simulink model "TosAeb"  (R2021a)
settings: Solver=FixedStepDiscrete  StartTime=0.0  StopTime=inf  FixedStep=0.01  RelTol=1e-3
undefined symbols (not defined in the file or in its folder): ACCModSts, AccSts1, AEB_ObjectMovingOrientation, AEB_ObjectMovingState, CN_BTCActive, CN_CurrentGearR, CN_CurrentGearR_DHT, CN_PTCActive, CN_VDCActive, CN_VSC_EspOff, CN_VSC_FCW_AEB_Error, CN_VSC_FCW_AEB_NoDispay, CN_VSC_FCW_AEB_PerfmDgdtn, CN_VSC_FCW_AEB_TempUnAvlbl, CN_VSC_VehStandstill, CNaccelAvoidMax, CNaccelAvoidMin, CNbrakePedalPress, CNcomfortStrLeftAccelMax, CNcomfortStrLeftAccelMin, CNcomfortStrRightAccelMax, CNcomfortStrRightAccelMin, CNDrvDoorOpen, CNDrvModeEco, CNDrvModeNorm, CNDrvModeOther, CNDrvModeSport, CNDrvSBRreminder, CNNull, CNobjInvalidID, CNPassDoorOpen, CNPassSBRreminder, CNPEBSAccelNegInf, CNPEBSAccelNull, CNPEBSTimeInf, CNPEBSTimeNull, CNSTOPMAX, CNSTOPNULL, CNsubjWidth, CNSwtOFF, CNSwtON, CNTTCMAX, CNTTCNULL, CNVehConfigAEBCycNone, CNVehConfigAEBNone, CNVehConfigAEBPedNone, CNVehConfigFCWNone, CNVehConfigJANone, CollisionSeverity, FALSE, FCWSnvty, JACycObjCalcInfoDefaultParams, JAPedObjCalcInfoDefaultParams, JAVehObjCalcInfoDefaultParams, latCycObjCalcInfoDefaultParams, latPedObjCalcInfoDefaultParams, longObjCalcInfoDefaultParams, ModSts, movingStatus, ObjectType ... (+1112)
libraries: simulink x3031, AEBSFunctionLib x50, Ta_Library x33, AEBSStateMachineLib x23, Mylibrary x12, simulink_extras x7, ObjSelect_lib x3, dspstat3 x2
(part 3 of 102)

== system TosAeb/FcwAebJa_Step_sys/ALG_AEBSystem/ALG_AEBOutput ==
  DiaManageInfo : Inport  OutDataTypeStr=Bus: BusDiagManageInfo
  VehCanInfo : Inport  Port=2
  vehConfigInfoOut : Inport  Port=3
  latPedCalcInfo : Inport  Port=4  OutDataTypeStr=Bus: BusLatPedObjCalcInfo
  latCycCalcInfo : Inport  Port=5  OutDataTypeStr=Bus: BusLatCycObjCalcInfo
  AEBSFuncOut : Inport  Port=6
  vehStateCalcInfo : Inport  Port=7
  longObjCalcInfo : Inport  Port=8
  AEBModStsFuncImpArb : Inport  Port=9
  AEBModRawSts : Inport  Port=10  OutDataTypeStr=Bus: BusAEBModRawSts
  latPedTgtCalcInfo : Inport  Port=11
  latCycTgtCalcInfo : Inport  Port=12
  AEBJAPedTgtCalcInfo : Inport  Port=13
  JAVehTgtCalcInfo : Inport  Port=14
  FCWSnvtyALGInfo : Inport  Port=15
  AEBS_BrkInfoCalc : Subsystem  TreatAsAtomicUnit=on
  AEBS_DVRInfoCalc : Subsystem  TreatAsAtomicUnit=on
  AEBS_IPHUTInfoCalc : Subsystem  TreatAsAtomicUnit=on
  AEB_EDR_JAPED_INFOMap : Subsystem  TreatAsAtomicUnit=on
  AEB_EDR_JAVEH_INFOMap : Subsystem  TreatAsAtomicUnit=on
  AEB_EDR_LATCYC_INFOMap : Subsystem  TreatAsAtomicUnit=on
  AEB_EDR_LATPED_INFOMap : Subsystem  TreatAsAtomicUnit=on
  AEB_EDR_LONOBJ_INFOMap : Subsystem  TreatAsAtomicUnit=on
  AEB_FUNC_STS_INFOMap : Subsystem  TreatAsAtomicUnit=on
  Bus Creator : BusCreator  Inputs=12
  Bus Selector : BusSelector  OutputSignals=AEBSFuncOut.ABALvl,AEBSFuncOut.ABATrigger,AEBModStsFuncImpArb.brkFuncImpArb.EBAFuncImpArb,AEBModRawSts.vehRawModSts.vehAEBpartRawModSts,AEBModRawSts.vehRawModSts.vehAEBfullRawModSts,AEBModRawSts.vehRawModSts.vehEBARawModSts,AEBModRawSts.latAEBRawModSts.pedAEBfullRawModSts,AEBModRawSts.latAEBRawModSts.cycAEBpartRawModSts,AEBModRawSts.latAEBRawModSts.cycAEBfullRawModSts,AEBModRawSts.JA_AEBRawModSts.JA_vehAEBRawModSts,AEBModRawSts.JA_AEBRawModSts.JA_pedAEBRawModSts,AEBSFuncOut.AEBTrigger,AEBSFuncOut.axTgtReqValue,AEBSFuncOut.acuteWarningTrigger,AEBSFuncOut.acuteWarningLvl,AEBSFuncOut.ABPTrigger,AEBModStsFuncImpArb.brkFuncImpArb.AEBpartFuncImpArb,AEBModStsFuncImpArb.brkFuncImpArb.AEBfullFuncImpArb,AEBModStsFuncImpArb.brkFuncImpArb.cycAEBpartFuncImpArb,AEBModStsFuncImpArb.brkFuncImpArb.cycAEBfullFuncImpArb,AEBModStsFuncImpArb.brkFuncImpArb.pedAEBfullFuncImpArb,AEBModStsFuncImpArb.brkFuncImpArb.JA_AEBfullFuncImpArb,AEBModStsFuncImpArb.brkFuncImpArb.FCTBFuncImpArb,AEBModRawSts.JA_AEBRawModSts.JA_cycAEBRawModSts  OutputAsBus=on
  Bus Selector1 : BusSelector  OutputSignals=vehConfigInfo.VehicleConfigFCW,vehConfigInfo.VehicleConfigAEB,longObjCalcInfo,vehConfigInfo.VehicleConfigAEBPed,latPedTgtCalcInfo,vehConfigInfo.VehicleConfigAEBCyc,latCycTgtCalcInfo,JAVehTgtCalcInfo,vehConfigInfo.VehConfigAEBJA,AEBJAPedTgtCalcInfo
  Bus Selector2 : BusSelector  OutputSignals=vehConfigInfo.VehicleConfigFCW,vehConfigInfo.VehicleConfigAEB,longObjCalcInfo
  Bus Selector3 : BusSelector  OutputSignals=vehConfigInfo.VehicleConfigAEBPed,latPedTgtCalcInfo
  Bus Selector4 : BusSelector  OutputSignals=vehConfigInfo.VehicleConfigAEBCyc,latCycTgtCalcInfo
  Bus Selector5 : BusSelector  OutputSignals=vehConfigInfo.VehConfigAEBJA,AEBJAPedTgtCalcInfo
  Bus Selector6 : BusSelector  OutputSignals=vehConfigInfo.VehConfigAEBJA,JAVehTgtCalcInfo
  Bus Selector7 : BusSelector  OutputSignals=AEBModStsFuncImpArb
  AEB_BRK_INFO : Outport  OutDataTypeStr=Bus: AEB_BRK_INFO_BUS
  AEB_DVR_INFO : Outport  Port=2  OutDataTypeStr=Bus: AEB_DVR_INFO_BUS
  AEB_IPHUT_INFO : Outport  Port=3  OutDataTypeStr=Bus: AEB_IPHUT_INFO_BUS
  AEB_EDR_LONOBJ_INFO : Outport  Port=4  OutDataTypeStr=Bus: AEB_EDR_LONOBJ_INFO_BUS
  AEB_EDR_LATPED_INFO : Outport  Port=5  OutDataTypeStr=Bus: AEB_EDR_LATPED_INFO_BUS
  AEB_EDR_LATCYC_INFO : Outport  Port=6  OutDataTypeStr=Bus: AEB_EDR_LATCYC_INFO_BUS
  AEB_EDR_JAPED_INFO : Outport  Port=7  OutDataTypeStr=Bus: AEB_EDR_JAPED_INFO_BUS
  AEB_EDR_JAVEH_INFO : Outport  Port=8  OutDataTypeStr=Bus: AEB_EDR_JAVEH_INFO_BUS
  AEB_FUNC_STS_INFO : Outport  Port=9  OutDataTypeStr=Bus: AEB_FUNC_STS_INFO_BUS
  wires:
  Bus Selector4/2 -> AEB_EDR_LATCYC_INFOMap/2(latCycTgtCalcInfo)  "<latCycTgtCalcInfo>"
  AEBSFuncOut/1 -> Bus Creator/4  "AEBSFuncOut"
  AEBJAPedTgtCalcInfo/1 -> Bus Creator/11  "AEBJAPedTgtCalcInfo"
  AEBModStsFuncImpArb/1 -> Bus Creator/8  "AEBModStsFuncImpArb"
  VehCanInfo/1 -> Bus Creator/2  "VehCanInfo"
  AEB_EDR_LATCYC_INFOMap/1(AEB_EDR_LATCYC_INFO) -> AEB_EDR_LATCYC_INFO/1
  JAVehTgtCalcInfo/1 -> Bus Creator/12  "JAVehTgtCalcInfo"
  latCycTgtCalcInfo/1 -> Bus Creator/10  "latCycTgtCalcInfo"
  Bus Selector1/1 -> AEBS_DVRInfoCalc/1(VehicleConfigFCW)  "<VehicleConfigFCW>"
  Bus Selector/1 -> AEBS_BrkInfoCalc/1(brkInfoInputSignals)
  Bus Selector1/8 -> AEBS_DVRInfoCalc/8(JAVehTgtCalcInfo)  "<JAVehTgtCalcInfo>"
  Bus Selector2/1 -> AEB_EDR_LONOBJ_INFOMap/1(VehicleConfigFCW)  "<VehicleConfigFCW>"
  AEB_FUNC_STS_INFOMap/1(AEB_FUNC_STS_INFO) -> AEB_FUNC_STS_INFO/1
  Bus Selector1/3 -> AEBS_DVRInfoCalc/3(longObjCalcInfo)  "<longObjCalcInfo>"
  latPedCalcInfo/1 -> AEBS_IPHUTInfoCalc/2(latPedCalcInfo)  "latPedCalcInfo"
  Bus Selector1/9 -> AEBS_DVRInfoCalc/9(VehConfigAEBJA)  "<VehConfigAEBJA>"
  Bus Selector7/1 -> AEB_FUNC_STS_INFOMap/1(AEBModStsFuncImpArb)  "<AEBModStsFuncImpArb>"
  vehStateCalcInfo/1 -> Bus Creator/5  "vehStateCalcInfo"
  Bus Creator/1 -> Bus Selector/1, Bus Selector1/1, Bus Selector2/1, Bus Selector3/1, Bus Selector4/1, Bus Selector5/1, Bus Selector6/1, Bus Selector7/1, AEBS_IPHUTInfoCalc/1(inputSignals)
  latPedTgtCalcInfo/1 -> Bus Creator/9  "latPedTgtCalcInfo"
  Bus Selector1/10 -> AEBS_DVRInfoCalc/10(AEBJAPedTgtCalcInfo)  "<AEBJAPedTgtCalcInfo>"
  Bus Selector2/2 -> AEB_EDR_LONOBJ_INFOMap/2(VehicleConfigAEB)  "<VehicleConfigAEB>"
  vehConfigInfoOut/1 -> Bus Creator/3  "vehConfigInfo"
  latCycCalcInfo/1 -> AEBS_IPHUTInfoCalc/3(latCycCalcInfo)  "latCycCalcInfo"
  AEBModRawSts/1 -> Bus Creator/7  "AEBModRawSts"
  longObjCalcInfo/1 -> Bus Creator/6  "longObjCalcInfo"
  AEBS_BrkInfoCalc/1(AEB_BRK_INFO) -> AEB_BRK_INFO/1
  AEB_EDR_LATPED_INFOMap/1(AEB_EDR_LATPED_INFO) -> AEB_EDR_LATPED_INFO/1
  Bus Selector5/2 -> AEB_EDR_JAPED_INFOMap/2(JaPedTgtCalcInfo)  "<AEBJAPedTgtCalcInfo>"
  AEB_EDR_LONOBJ_INFOMap/1(AEB_EDR_LONOBJ_INFO) -> AEB_EDR_LONOBJ_INFO/1
  AEB_EDR_JAVEH_INFOMap/1(AEB_EDR_JAVEH_INFO) -> AEB_EDR_JAVEH_INFO/1
  Bus Selector1/4 -> AEBS_DVRInfoCalc/4(VehicleConfigAEBPed)  "<VehicleConfigAEBPed>"
  Bus Selector1/5 -> AEBS_DVRInfoCalc/5(latPedTgtCalcInfo)  "<latPedTgtCalcInfo>"
  Bus Selector1/7 -> AEBS_DVRInfoCalc/7(latCycTgtCalcInfo)  "<latCycTgtCalcInfo>"
  Bus Selector2/3 -> AEB_EDR_LONOBJ_INFOMap/3(longObjCalcInfo)  "<longObjCalcInfo>"
  AEBS_IPHUTInfoCalc/1(AEB_IPHUT_INFO) -> AEB_IPHUT_INFO/1
  Bus Selector6/1 -> AEB_EDR_JAVEH_INFOMap/1(VehConfigAEBJA)  "<VehConfigAEBJA>"
  DiaManageInfo/1 -> Bus Creator/1  "DiaManageInfo"
  Bus Selector5/1 -> AEB_EDR_JAPED_INFOMap/1(VehConfigAEBJA)  "<VehConfigAEBJA>"
  Bus Selector4/1 -> AEB_EDR_LATCYC_INFOMap/1(VehicleConfigAEBCyc)  "<VehicleConfigAEBCyc>"
  Bus Selector3/2 -> AEB_EDR_LATPED_INFOMap/2(latPedTgtCalcInfo)  "<latPedTgtCalcInfo>"
  Bus Selector6/2 -> AEB_EDR_JAVEH_INFOMap/2(JaVehTgtCalcInfo)  "<JAVehTgtCalcInfo>"
  AEBS_DVRInfoCalc/1(AEB_DVR_INFO) -> AEB_DVR_INFO/1
  Bus Selector3/1 -> AEB_EDR_LATPED_INFOMap/1(VehicleConfigAEBPed)  "<VehicleConfigAEBPed>"
  AEB_EDR_JAPED_INFOMap/1(AEB_EDR_JAPED_INFO) -> AEB_EDR_JAPED_INFO/1
  Bus Selector1/6 -> AEBS_DVRInfoCalc/6(VehicleConfigAEBCyc)  "<VehicleConfigAEBCyc>"
  Bus Selector1/2 -> AEBS_DVRInfoCalc/2(VehicleConfigAEB)  "<VehicleConfigAEB>"
  FCWSnvtyALGInfo/1 -> AEBS_IPHUTInfoCalc/4(FCWSnvtyALGInfo)  "JAVehTgtCalcInfo"

== system TosAeb/AEB_Objsel/TA_InfoRebuild/TAObjInfoCalc/For Each Subsystem ==
  IDForEach : Inport  Partition=on  PartitionDimension=1  PartitionWidth=1  PartitionOffset=0
  Cam_laneLeftPortion : Inport  Port=2
  Cam_laneAssociateConf : Inport  Port=3
  Cam_laneRightPortion : Inport  Port=4
  Cam_laneLeftConf : Inport  Port=5
  Cam_laneRightConf : Inport  Port=6
  Cam_objIntention : Inport  Port=7
  Cam_objIntentionProb : Inport  Port=8
  Cam_laneAssign : Inport  Port=9
  Cam_ObsPosX_s : Inport  Port=10
  Cam_ObsPosY_s : Inport  Port=11
  Cam_ObsAccY_s : Inport  Port=12
  Cam_ObsAccX_s : Inport  Port=13
  Cam_ObsVelY_s : Inport  Port=14
  Cam_ObsVelX_s : Inport  Port=15
  Cam_ObsID_u8 : Inport  Port=16
  Cam_ObsValid_u8 : Inport  Port=17
  Road_Info : Inport  Port=18
  For Each : ForEach  DisableCoverage=on  ShowIterationIndex=on
  ForEachInput : Subsystem
  Info_rebuild : Subsystem
  Terminator : Terminator
  vxRel : Outport  ConcatenationDimension=1
  vyRel : Outport  Port=2  ConcatenationDimension=1
  vxRelStd : Outport  Port=3  ConcatenationDimension=1
  vyRelStd : Outport  Port=4  ConcatenationDimension=1
  axRel : Outport  Port=5  ConcatenationDimension=1
  ayRel : Outport  Port=6  ConcatenationDimension=1
  axRelStd : Outport  Port=7  ConcatenationDimension=1
  ayRelStd : Outport  Port=8  ConcatenationDimension=1
  azimuthAngle : Outport  Port=9  ConcatenationDimension=1
  azimuthAngleStd : Outport  Port=10  ConcatenationDimension=1
  objLatDist2LaneCenter : Outport  Port=11  ConcatenationDimension=1
  objLatDist2EgoCenter : Outport  Port=12  ConcatenationDimension=1
  objPosition : Outport  Port=13  ConcatenationDimension=1
  wires:
  Cam_objIntentionProb/1 -> ForEachInput/8(Cam_objIntentionProb)
  Cam_laneRightConf/1 -> ForEachInput/6(Cam_laneRightConf)
  IDForEach/1 -> Terminator/1
  Info_rebuild/12(objLatDist2EgoCenter) -> objLatDist2EgoCenter/1
  Info_rebuild/10(azimuthAngleStd) -> azimuthAngleStd/1
  For Each/1 -> ForEachInput/1(Index)
  Cam_laneLeftPortion/1 -> ForEachInput/2(Cam_laneLeftPortion)
  Cam_laneAssociateConf/1 -> ForEachInput/3(Cam_laneAssociateConf)
  Cam_laneAssign/1 -> ForEachInput/9(Cam_laneAssign)
  Cam_objIntention/1 -> ForEachInput/7(Cam_objIntention)
  Cam_laneRightPortion/1 -> ForEachInput/4(Cam_laneRightPortion)
  Cam_laneLeftConf/1 -> ForEachInput/5(Cam_laneLeftConf)
  Cam_ObsPosX_s/1 -> ForEachInput/10(Cam_ObsPosX_s)
  Cam_ObsPosY_s/1 -> ForEachInput/11(Cam_ObsPosY_s)
  Cam_ObsAccY_s/1 -> ForEachInput/12(Cam_ObsAccY_s)
  Cam_ObsAccX_s/1 -> ForEachInput/13(Cam_ObsAccX_s)
  Cam_ObsVelY_s/1 -> ForEachInput/14(Cam_ObsVelY_s)
  Cam_ObsVelX_s/1 -> ForEachInput/15(Cam_ObsVelX_s)
  Info_rebuild/11(objLatDist2LaneCenter) -> objLatDist2LaneCenter/1
  ForEachInput/1(Obj_Infomation) -> Info_rebuild/1(Obj_Infomation)
  Road_Info/1 -> Info_rebuild/2(Road_Info)
  Info_rebuild/1(vxRel) -> vxRel/1
  Info_rebuild/4(vyRelStd) -> vyRelStd/1
  Info_rebuild/2(vyRel) -> vyRel/1
  Info_rebuild/3(vxRelStd) -> vxRelStd/1
  Info_rebuild/7(axRelStd) -> axRelStd/1
  Info_rebuild/5(axRel) -> axRel/1
  Info_rebuild/6(ayRel) -> ayRel/1
  Info_rebuild/8(ayRelStd) -> ayRelStd/1
  Info_rebuild/9(azimuthAngle) -> azimuthAngle/1
  Info_rebuild/13(objPosition) -> objPosition/1
  Cam_ObsValid_u8/1 -> ForEachInput/17(Cam_ObsValid_u8)
  Cam_ObsID_u8/1 -> ForEachInput/16(Cam_ObsID_u8)

== system TosAeb/AEB_Objsel/TA_InfoRebuild/TAObjInfoCalc/Signal_Rebuild ==
  vxRel : Inport
  vyRel : Inport  Port=2
  vxRelStd : Inport  Port=3
  vyRelStd : Inport  Port=4
  axRel : Inport  Port=5
  ayRel : Inport  Port=6
  axRelStd : Inport  Port=7
  ayRelStd : Inport  Port=8
  azimuthAngle : Inport  Port=9
  azimuthAngleStd : Inport  Port=10
  objLatDist2LaneCenter : Inport  Port=11
  objLatDist2EgoCenter : Inport  Port=12
  objPosition : Inport  Port=13
  Bus Creator : BusCreator  Inputs=13  OutDataTypeStr=Bus: Objrebuild_Bus  NonVirtualBus=on
  Objrebuild_Struct : Outport
  wires:
  vxRel/1 -> Bus Creator/1  "vxRel"
  vxRelStd/1 -> Bus Creator/3  "vxRelStd"
  vyRel/1 -> Bus Creator/2  "vyRel"
  axRel/1 -> Bus Creator/5  "axRel"
  ayRel/1 -> Bus Creator/6  "ayRel"
  vyRelStd/1 -> Bus Creator/4  "vyRelStd"
  azimuthAngleStd/1 -> Bus Creator/10  "azimuthAngleStd"
  ayRelStd/1 -> Bus Creator/8  "ayRelStd"
  azimuthAngle/1 -> Bus Creator/9  "azimuthAngle"
  axRelStd/1 -> Bus Creator/7  "axRelStd"
  objLatDist2LaneCenter/1 -> Bus Creator/11  "objLatDist2LaneCenter"
  objLatDist2EgoCenter/1 -> Bus Creator/12  "objLatDist2EgoCenter"
  objPosition/1 -> Bus Creator/13  "objPosition"
  Bus Creator/1 -> Objrebuild_Struct/1

== system TosAeb/AEB_Objsel/TA_Processor/AEB_ObjSelect/TargetInfoCheck ==
  TA_ObjSumInfo : Inport
  VehSelfData : Inport  Port=2
  PathEstimn : Inport  Port=3
  AccTgtIdn : Inport  Port=4
  Data_precess : Subsystem  TreatAsAtomicUnit=on
  Each_Set : Subsystem
  Signal Conversion : SignalConversion  OverrideOpt=off
  Signal Conversion1 : SignalConversion  OverrideOpt=off
  Signal Conversion2 : SignalConversion  OverrideOpt=off
  Terminator : Terminator
  Terminator1 : Terminator
  Terminator2 : Terminator
  TA_ObjSumInfo_P : Outport
  VehSelfData_P : Outport  Port=2
  AccTgtIdn_P : Outport  Port=3
  Boudingbox_Calculate : Outport  Port=4
  Obj_Coef : Outport  Port=5
  TTI : Outport  Port=6
  LatObj_Valid : Outport  Port=7
  Lat_ObjIntersect : Outport  Port=8
  Lat_TTR : Outport  Port=9
  Overlap_Flg : Outport  Port=10
  JA_TTRAssign : Outport  Port=11
  wires:
  Each_Set/1(TA_ObjID) -> Data_precess/1(TA_ObjID)  "Meas_ObjID"
  TA_ObjSumInfo/1 -> Each_Set/1(TA_ObjSumInfo), [TA_ObjSumInfo]
  Each_Set/15(TA_ObjVxAbs) -> Data_precess/15(TA_ObjVxAbs)  "Meas_ObjVxabs"
  VehSelfData/1 -> [VehSelfData], Data_precess/29(VehSelfData)
  Each_Set/10(TA_ObjDyStd) -> Data_precess/10(TA_ObjDyStd)  "Meas_ObjDyStd"
  Each_Set/24(TA_ObjConfidence) -> Data_precess/24(TA_ObjConfidence)  "Meas_ObjConfidence"
  Each_Set/13(TA_ObjVxStd) -> Data_precess/13(TA_ObjVxStd)  "Meas_ObjVxStd"
  Each_Set/4(TA_ObjOrientation) -> Data_precess/4(TA_ObjOrientation)  "Meas_ObjOrientation"
  Each_Set/26(TA_ObjHeadAngle) -> Data_precess/26(TA_ObjHeadAngle)  "Meas_ObjHeadAngle"
  Each_Set/27(TA_ObjHeadAngleStd) -> Data_precess/27(TA_ObjHeadAngleStd)
  Each_Set/14(TA_ObjVyStd) -> Data_precess/14(TA_ObjVyStd)  "Meas_ObjVyStd"
  Each_Set/18(TA_ObjWidth) -> Data_precess/18(TA_ObjWidth)  "Meas_ObjWidth"
  Each_Set/3(TA_ObjState) -> Data_precess/3(TA_ObjState)  "Meas_ObjState"
  Each_Set/23(TA_ObjAyAbs) -> Data_precess/23(TA_ObjAyAbs)  "Meas_ObjAyabs"
  Each_Set/22(TA_ObjAxAbs) -> Data_precess/22(TA_ObjAxAbs)  "Meas_ObjAxabs"
  Each_Set/5(TA_ObjfusType) -> Data_precess/5(TA_ObjfusType)
  Each_Set/21(TA_ObjAy) -> Data_precess/21(TA_ObjAy)  "Meas_ObjAy"
  Each_Set/17(TA_ObjLength) -> Data_precess/17(TA_ObjLength)  "Meas_ObjLength"
  Each_Set/19(TA_ObjHight) -> Data_precess/19(TA_ObjHight)
  Each_Set/25(TA_ObjCenterAngle) -> Data_precess/25(TA_ObjCenterAngle)
  Each_Set/20(TA_ObjAx) -> Data_precess/20(TA_ObjAx)  "Meas_ObjAx"
  Each_Set/6(TA_ObjLaneAssign) -> Data_precess/6(TA_ObjLaneAssign)
  [AccTgtIdn] -> AccTgtIdn_P/1
  Each_Set/12(TA_ObjVy) -> Data_precess/12(TA_ObjVy)  "Meas_ObjVy"
  [VehSelfData] -> VehSelfData_P/1
  Each_Set/7(TA_ObjDx) -> Data_precess/7(TA_ObjDx)  "Meas_ObjDx"
  Each_Set/2(TA_ObjType) -> Data_precess/2(TA_ObjType)  "Meas_ObjType"
  Data_precess/2(Obj_Coef) -> Signal Conversion1/1  "Meas_ObjCoef"
  Data_precess/5(Lat_ObjIntersect) -> Lat_ObjIntersect/1
  Data_precess/7(Overlap_Flg) -> Overlap_Flg/1
  [TA_ObjSumInfo] -> TA_ObjSumInfo_P/1
  Each_Set/8(TA_ObjDy) -> Data_precess/8(TA_ObjDy)  "Meas_ObjDy"
  Data_precess/1(Boudingbox_Calculate) -> Signal Conversion/1  "Meas_Boudingbox_Calculate"
  Each_Set/9(TA_ObjDxStd) -> Data_precess/9(TA_ObjDxStd)  "Meas_ObjDxStd"
  AccTgtIdn/1 -> [AccTgtIdn]
  Data_precess/3(TTI) -> Signal Conversion2/1  "Meas_LongTTI"
  Each_Set/11(TA_ObjVx) -> Data_precess/11(TA_ObjVx)  "Meas_ObjVx"
  Data_precess/6(Lat_TTR) -> Lat_TTR/1
  Data_precess/4(LatObj_Valid) -> LatObj_Valid/1
  PathEstimn/1 -> Data_precess/30(PathEstimn)
  Each_Set/28(IDForeach) -> Data_precess/28(IDForeach)
  Data_precess/8(JA_TTRAssign) -> JA_TTRAssign/1
  Signal Conversion/1 -> Boudingbox_Calculate/1
  Signal Conversion1/1 -> Obj_Coef/1
  Signal Conversion2/1 -> TTI/1
  Data_precess/9(Utility) -> Terminator/1  "Meas_Utility"
  Each_Set/16(TA_ObjVyAbs) -> Data_precess/16(TA_ObjVyAbs)  "Meas_ObjVyabs"
  Data_precess/10(Meas_WeightCoef_1) -> Terminator1/1  "Meas_WeightCoef_1"
  Data_precess/11(Meas_WeightCoef_2) -> Terminator2/1  "Meas_WeightCoef_2"

== system TosAeb/AEB_Objsel/TA_Processor/AEB_ObjSelect/TargetSelect ==
  TA_ObjInfo : Inport
  VehSelfData : Inport  Port=2
  AccTgtIdn : Inport  Port=3
  Boudingbox_Calculate : Inport  Port=4
  Obj_Coef : Inport  Port=5
  TTI : Inport  Port=6
  LatObj_Valid : Inport  Port=7
  Lat_ObjIntersect : Inport  Port=8
  Lat_TTR : Inport  Port=9
  Overlap_Flg : Inport  Port=10
  JA_TTRAssign : Inport  Port=11
  JA_ObjSelect : Subsystem  Commented=on
  Lat_ObjSelect : Subsystem
  Long_ObjSelect : Subsystem
  Signal Conversion : SignalConversion  OverrideOpt=off
  Long_ObjSortNum : Outport
  Long_ObjID : Outport  Port=2
  Lat_ObjSortNum : Outport  Port=3
  Lat_ObjID : Outport  Port=4
  Ta_Info : Outport  Port=5
  Long_TTC : Outport  Port=6
  Lat_TTC : Outport  Port=7
  JA_TTC : Outport  Port=8
  wires:
  TA_ObjInfo/1 -> [TA_ObjInfo], Long_ObjSelect/1(TA_ObjInfo)
  VehSelfData/1 -> Long_ObjSelect/2(VehSelfData)
  Boudingbox_Calculate/1 -> Long_ObjSelect/3(Boudingbox_Calculate)
  Obj_Coef/1 -> Long_ObjSelect/4(Obj_Coef)
  TTI/1 -> Long_ObjSelect/5(TTI)
  AccTgtIdn/1 -> Long_ObjSelect/6(AccTgtIdn)
  Long_ObjSelect/2(Long_ObjID) -> Long_ObjID/1
  Long_ObjSelect/1(Long_ObjSortNum) -> Long_ObjSortNum/1
  Overlap_Flg/1 -> Long_ObjSelect/7(Overlap_Rate)
  Long_ObjSelect/3(long_TTC) -> Long_TTC/1
  Lat_ObjSelect/1(Lat_ObjSortNum) -> Lat_ObjSortNum/1
  [Lat_TTC] -> Lat_TTC/1
  [TA_ObjInfo] -> Lat_ObjSelect/4(TA_ObjInfo)
  Lat_ObjSelect/2(Lat_ObjID) -> Lat_ObjID/1
  Lat_ObjIntersect/1 -> Lat_ObjSelect/2(Lat_ObjIntersect)
  Lat_TTR/1 -> Lat_ObjSelect/3(Lat_TTR), [Lat_TTC]
  LatObj_Valid/1 -> Lat_ObjSelect/1(LatObj_Valid)
  [TA_ObjInfo] -> Ta_Info/1
  JA_TTRAssign/1 -> Signal Conversion/1
  Signal Conversion/1 -> JA_TTC/1

== system TosAeb/AEB_Objsel/TA_Processor/PreProcessor/ExtractAccTargetIdIfAccActive ==
  ACC_Infout : Inport
  Bus Selector : BusSelector  OutputSignals=ACC_TargetID,ACC_Mode
  ConfirmAccId : Subsystem
  Constant : Constant  Value=uint8(0)  OutDataTypeStr=uint8
  Constant1 : Constant  OutDataTypeStr=uint8
  Constant2 : Constant  Value=2  OutDataTypeStr=uint8
  Constant3 : Constant  Value=3  OutDataTypeStr=uint8
  Enumerated Constant3 : Subsystem  Commented=on  DialogController=Simulink.DDGSource  mask(Enumerated Constant){OutDataTypeStr=Enum: AccSts1, Value=AccSts1.Stop, SampleTime=inf}
      mask prompts: OutDataTypeStr "Simulink:masks:OutputDataType_MP"; Value "Simulink:masks:Value_MP"; SampleTime "Simulink:masks:SampleTime_MP"
      mask init:
      | % NOTE:
      | % Value is not evaluated to avoid caching an instance
      | % of the MCOS class by the mask infrastructure.
      | 
      | % Get string to use for display purposes
      | enumConstDispStr = Value;
      | if isempty(enumConstDispStr)
      |   enumConstDispStr = '???';
      | end
      | 
      | % Check data type & value
      | enumconst_cb('MaskInit', OutDataTypeStr, slResolve(Value, gcb));
      | 
      | % Set mask parameter values directly on underlying block
      | % and clear mask workspace variables to prevent underlying
      | % blocks from resolving to unevaluated mask variables.
      | set_param([gcb, '/Constant'], ...
      |     'OutDataTypeStr', OutDataTypeStr, ...
      |     'SampleTime',     SampleTime, ...
      |     'Value',          Value);
      | clear OutDataTypeStr SampleTime Value;
      mask Display: disp(enumConstDispStr)
  Enumerated Constant4 : Subsystem  Commented=on  DialogController=Simulink.DDGSource  mask(Enumerated Constant){OutDataTypeStr=Enum: AccSts1, Value=AccSts1.StopTmp, SampleTime=inf}
      mask prompts: OutDataTypeStr "Simulink:masks:OutputDataType_MP"; Value "Simulink:masks:Value_MP"; SampleTime "Simulink:masks:SampleTime_MP"
      mask init: same as TosAeb/AEB_Objsel/TA_Processor/PreProcessor/ExtractAccTargetIdIfAccActive/Enumerated Constant3
      mask Display: disp(enumConstDispStr)
  Enumerated Constant5 : Subsystem  Commented=on  DialogController=Simulink.DDGSource  mask(Enumerated Constant){OutDataTypeStr=Enum: AccSts1, Value=AccSts1.Actv, SampleTime=inf}
      mask prompts: OutDataTypeStr "Simulink:masks:OutputDataType_MP"; Value "Simulink:masks:Value_MP"; SampleTime "Simulink:masks:SampleTime_MP"
      mask init: same as TosAeb/AEB_Objsel/TA_Processor/PreProcessor/ExtractAccTargetIdIfAccActive/Enumerated Constant3
      mask Display: disp(enumConstDispStr)
  Logical Operator : Logic  Operator=OR  Inputs=3  AllPortsSameDT=off  OutDataTypeStr=boolean
  Relational Operator : RelationalOperator  Operator===  OutDataTypeStr=boolean  RndMeth=Nearest
  Relational Operator1 : RelationalOperator  Operator===  OutDataTypeStr=boolean  RndMeth=Nearest
  Relational Operator2 : RelationalOperator  Operator===  OutDataTypeStr=boolean  RndMeth=Nearest
  Switch : Switch  Criteria=u2 ~= 0
  AccTgtIdn : Outport  SampleTime=0.02
  wires:
  ConfirmAccId/1(Confirmed_ AccP0Id) -> Switch/1
  Bus Selector/1 -> ConfirmAccId/1(Acc_TaP0Id)  "<ACC_TargetID>"
  Switch/1 -> AccTgtIdn/1  "AccTgtIdn"
  Constant/1 -> Switch/3
  Logical Operator/1 -> Switch/2
  Relational Operator2/1 -> Logical Operator/3
  Relational Operator1/1 -> Logical Operator/2
  Relational Operator/1 -> Logical Operator/1
  Constant3/1 -> Relational Operator2/2
  Constant2/1 -> Relational Operator1/2
  Constant1/1 -> Relational Operator/2
  Bus Selector/2 -> Relational Operator2/1, Relational Operator1/1, Relational Operator/1  "<ACC_Mode>"
  ACC_Infout/1 -> Bus Selector/1

== system TosAeb/AEB_Objsel/TA_Processor/PreProcessor/ObjectPrecessor ==
  VehSelfIn : Inport
  Fus_TargetBus : Inport  Port=2  OutDataTypeStr=Bus: TA_ObjProInfo
  TAObjInfoCalc : Subsystem  TreatAsAtomicUnit=on
  TA_ObjInfo : Outport
  wires:
  VehSelfIn/1 -> TAObjInfoCalc/1(VehSelfIn)
  TAObjInfoCalc/1(TA_ObjInfo) -> TA_ObjInfo/1
  Fus_TargetBus/1 -> TAObjInfoCalc/2(Fus_TargetBus)

== system TosAeb/AEB_Objsel/TA_Processor/PreProcessor/VehiclePreProcessor ==
  VehSelfIn : Inport
  Bus Creator1 : BusCreator  Inputs=5
  Bus Selector : BusSelector  OutputSignals=AEB_Intervention_ESP
  Bus Selector1 : BusSelector  OutputSignals=ABS_VehicleSpeed,AX1
  CalculateCurvature : Subsystem
  LongitudinalAccelerationWithoutCMbB : Subsystem
  PathEstimn_Calculate : Subsystem
  VehSelfData : Outport
  PathEstimn : Outport  Port=2
  wires:
  CalculateCurvature/2(CurvatureRate) -> [CurvatureRate], Bus Creator1/3
  CalculateCurvature/1(Curvature) -> Bus Creator1/2, [Curvature]
  Bus Creator1/1 -> VehSelfData/1  "VehSelfData"
  VehSelfIn/1 -> Bus Selector1/1, Bus Selector/1, LongitudinalAccelerationWithoutCMbB/1(VehSelf), CalculateCurvature/1(VehSelf), Bus Creator1/1
  Bus Selector/1 -> LongitudinalAccelerationWithoutCMbB/2(ActtnDataFromCMbB)  "<AEB_Intervention_ESP>"
  PathEstimn_Calculate/1(PahEstimn) -> PathEstimn/1
  Bus Selector1/1 -> PathEstimn_Calculate/1(Veh_LgtSpd_mps)  "<ABS_VehicleSpeed>"
  Bus Selector1/2 -> PathEstimn_Calculate/2(Veh_LgtAccel_mps2)  "<AX1>"
  [Curvature] -> PathEstimn_Calculate/3(Curvature)
  [CurvatureRate] -> PathEstimn_Calculate/4(CurvatureRate)
  LongitudinalAccelerationWithoutCMbB/1(DrvrALgt) -> Bus Creator1/5
  CalculateCurvature/3(CurvatureRadius) -> Bus Creator1/4

== system TosAeb/AEB_Objsel/TA_Processor/TA_Check/TA_LatCheck ==
  Lat_ObjSortNum : Inport
  Lat_ObjID : Inport  Port=2
  Lat_TTC : Inport  Port=3
  Bus Creator : BusCreator  Inputs=3  OutDataTypeStr=Bus: objLat_Bus  NonVirtualBus=on
  Display : Display
  Display1 : Display
  Display2 : Display
  For Iterator Subsystem : Subsystem  TreatAsAtomicUnit=on
  Lat_Check : MATLAB Function  TreatAsAtomicUnit=on
  Signal Conversion : SignalConversion  OverrideOpt=off
  Signal Conversion1 : SignalConversion  OverrideOpt=off
  Signal Conversion2 : SignalConversion  OverrideOpt=off
  ObjLat_Bus : Outport
  wires:
  Signal Conversion/1 -> Display/1, Bus Creator/2  "ObjSortNum_Lat"
  Signal Conversion1/1 -> Bus Creator/1  "ID_Lat"
  Signal Conversion2/1 -> Bus Creator/3  "TTC_Lat"
  Bus Creator/1 -> ObjLat_Bus/1
  Lat_ObjSortNum/1 -> Lat_Check/2(Lat_ObjSortNum), For Iterator Subsystem/1(Lat_ObjSortNum)
  Lat_ObjID/1 -> Lat_Check/1(Lat_ObjID)
  Lat_Check/1(ID) -> Signal Conversion1/1
  Lat_Check/2(Num) -> Signal Conversion/1
  Lat_Check/3(Ttc) -> Signal Conversion2/1
  Lat_TTC/1 -> Display1/1, For Iterator Subsystem/2(Lat_TTC)
  For Iterator Subsystem/1(ttc) -> Display2/1, Lat_Check/3(Lat_TTC)

== system TosAeb/AEB_Objsel/TA_Processor/TA_Check/TA_LongCheck ==
  Long_ObjSortNum : Inport
  Long_ObjID : Inport  Port=2  OutDataTypeStr=uint8
  Long_TTC : Inport  Port=3
  TA_Info : Inport  Port=4
  Bus Creator : BusCreator  Inputs=3  OutDataTypeStr=Bus: ObjLong_Bus  NonVirtualBus=on
  Bus Selector1 : BusSelector  OutputSignals=TA_ObjID
  Constant : Constant  Value=0  OutDataTypeStr=uint8
  Constant1 : Constant  Value=0  OutDataTypeStr=uint8
  Constant2 : Constant  Value=0  OutDataTypeStr=boolean
  Constant3 : Constant  Value=0  OutDataTypeStr=single
  Constant4 : Constant  Value=0  OutDataTypeStr=boolean
  Constant5 : Constant  Value=0  OutDataTypeStr=single
  ID_Confirm : MATLAB Function  TreatAsAtomicUnit=on
  Signal Conversion : SignalConversion  OverrideOpt=off
  Signal Conversion1 : SignalConversion  OverrideOpt=off
  Signal Conversion2 : SignalConversion  OverrideOpt=off
  ObjLong_Bus : Outport
  wires:
  Long_ObjID/1 -> ID_Confirm/8(Long_ObjID)
  Long_TTC/1 -> ID_Confirm/9(Long_TTC)
  Long_ObjSortNum/1 -> ID_Confirm/10(Long_ObjSortNum)
  Signal Conversion/1 -> Bus Creator/1  "ID_Long"
  Signal Conversion1/1 -> Bus Creator/2  "ObjSortNum_Long"
  Signal Conversion2/1 -> Bus Creator/3  "TTCLong"
  TA_Info/1 -> Bus Selector1/1
  Bus Selector1/1 -> ID_Confirm/1(Obs_ID)  "<TA_ObjID>"
  Constant/1 -> ID_Confirm/2(CIPV_ID)
  Constant1/1 -> ID_Confirm/3(MPC_ID)
  Constant2/1 -> ID_Confirm/4(CIPV_TtcValid)
  Constant3/1 -> ID_Confirm/5(CIPV_Ttc)
  Constant4/1 -> ID_Confirm/6(MPC_TtcValid)
  Constant5/1 -> ID_Confirm/7(MPC_Ttc)
  Bus Creator/1 -> ObjLong_Bus/1
  ID_Confirm/1(ID) -> Signal Conversion/1  "Meas_ID_Long"
  ID_Confirm/2(ObjSortNum) -> Signal Conversion1/1  "Meas_ObjSortNum_Long"
  ID_Confirm/3(TTC) -> Signal Conversion2/1  "Meas_TTCLong"

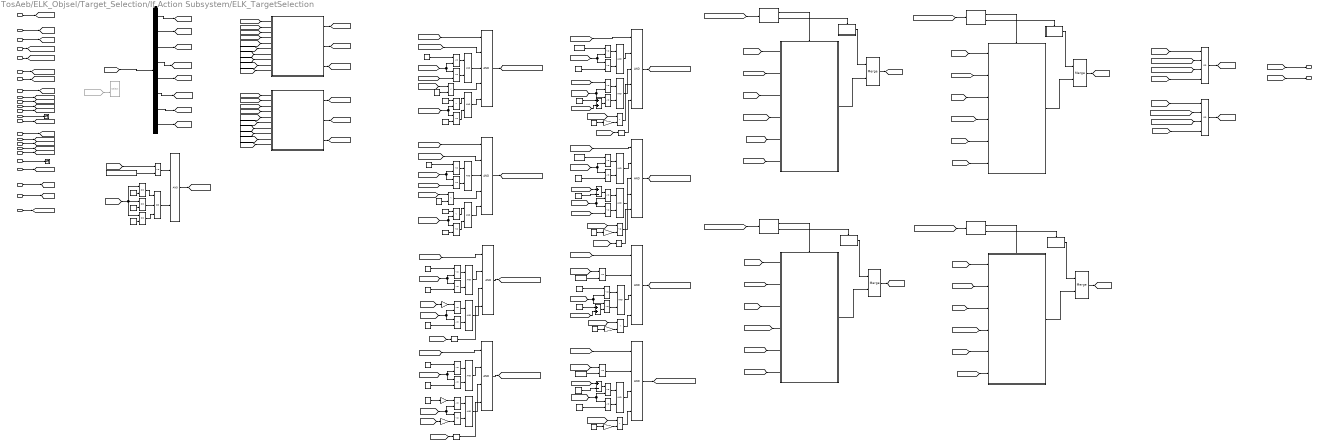
== system TosAeb/ELK_Objsel/Target_Selection/If Action Subsystem/ELK_TargetSelection ==
  note: 改">"为<=
  note: 去掉-号
  note: 需通过标定确定，目标纵向与本车有重叠时的纵向距离范围
  note: 需通过标定确定，目标纵向与本车有重叠时的纵向距离范围
  note: 去掉-号
  ForIterator_enum : Inport
  Objs_all : Inport  Port=2
  Host_Veh_Width : Inport  Port=3
  left_boundary_lat_rate_filtered : Inport  Port=4
  right_boundary_lat_rate_filtered : Inport  Port=5
  ELK_LeftLine_Valid_Flag : Inport  Port=6
  ELK_RightLine_Valid_Flag : Inport  Port=7
  left_line_width : Inport  Port=8
  a0_LeftLine : Inport  Port=9
  a1_LeftLine : Inport  Port=10
  a2_LeftLine : Inport  Port=11
  a3_LeftLine : Inport  Port=12
  SP_LeftLine : Inport  Port=13
  EP_LeftLine : Inport  Port=14
  right_line_width : Inport  Port=15
  a0_RightLine : Inport  Port=16
  a1_RightLine : Inport  Port=17
  a2_RightLine : Inport  Port=18
  a3_RightLine : Inport  Port=19
  SP_RightLine : Inport  Port=20
  EP_RightLine : Inport  Port=21
  vxAbsObj : Inport  Port=22
  vyAbsObj : Inport  Port=23
  TTC_Calculated : Inport  Port=24
  Add : Sum
  Add1 : Sum  Inputs=+-
  Add2 : Sum  Inputs=+-
  Add3 : Sum
  Bus Selector1 : BusSelector  OutputSignals=objID,objType,objWidth,dxCenter,dyCenter,vxRel,vyRel,movingStatus
  Compare To Constant{,1..3} (4 alike) : simulink/Logic and Bit Operations/Compare To Constant  relop=~=  const=8  OutDataTypeStr=boolean
  Constant{89,93,97,100} (4 alike) : Constant  Value=10
  Constant{62,66,68..69,73,78,81,83,99,101} (10 alike) : Constant  Value=0
  Constant70 : Constant  Value=4
  Constant{71,82,88,92} (4 alike) : Constant  Value=-5
  Constant72 : Constant  Value=ObjSelect_CalELKInvalidObjID
  Constant{74..75,90,94..95} (5 alike) : Constant  Value=3
  Constant76 : Constant  Value=4
  Constant77 : Constant  Value=5
  Constant84 : Constant  Value=4
  Constant85 : Constant  Value=2
  Constant86 : Constant  Value=2
  Constant{87,91,96,98} (4 alike) : Constant  Value=-12.856
  From37 : From  Commented=on  GotoTag=ForIterator_enum
  Gain : Gain  Gain=0.5
  Gain1 : Gain  Gain=-1
  Gain2 : Gain  Gain=-0.5
  Gain{3..5,7} (4 alike) : Gain  Gain=1/3.6
  If Action Subsystem : Subsystem  TreatAsAtomicUnit=on
  If Action Subsystem1 : Subsystem  TreatAsAtomicUnit=on
  If Action Subsystem4 : Subsystem  TreatAsAtomicUnit=on
  If Action Subsystem5 : Subsystem  TreatAsAtomicUnit=on
  If Action Threat_Assessment_Left : Subsystem  TreatAsAtomicUnit=on
  If Action Threat_Assessment_Left1 : Subsystem  TreatAsAtomicUnit=on
  If Action Threat_Assessment_Right : Subsystem  TreatAsAtomicUnit=on
  If Action Threat_Assessment_Right1 : Subsystem  TreatAsAtomicUnit=on
  If{1,4..6} (4 alike) : If  IfExpression=u1 ~= 0
  Left : Subsystem
  Logical Operator{1,9,15..16} (4 alike) : Logic  Inputs=5  AllPortsSameDT=off  OutDataTypeStr=boolean
  Logical Operator{3..4,6..8,10..11,13..14,17..20,23..24} (15 alike) : Logic  AllPortsSameDT=off  OutDataTypeStr=boolean
  Logical Operator12 : Logic  Operator=OR  Inputs=3  AllPortsSameDT=off  OutDataTypeStr=boolean
  Logical Operator{2,5,21..22} (4 alike) : Logic  Inputs=4  AllPortsSameDT=off  OutDataTypeStr=boolean
  Logical Operator27 : Logic  Operator=OR  Inputs=4  AllPortsSameDT=off  OutDataTypeStr=boolean
  Logical Operator28 : Logic  Operator=OR  Inputs=4  AllPortsSameDT=off  OutDataTypeStr=boolean
  Merge{,1..3} (4 alike) : Merge
  Relational Operator{,1..3,6..8,15..20,25,27..33,35..37,39..40} (26 alike) : RelationalOperator  Operator=<=  OutDataTypeStr=boolean  RndMeth=Simplest
  Relational Operator10 : RelationalOperator  Operator===  OutDataTypeStr=boolean  RndMeth=Simplest
  Relational Operator12 : RelationalOperator  Operator===  OutDataTypeStr=boolean  RndMeth=Simplest
  Relational Operator13 : RelationalOperator  Operator===  OutDataTypeStr=boolean  RndMeth=Simplest
  Relational Operator14 : RelationalOperator  Operator=<  OutDataTypeStr=boolean  RndMeth=Nearest
  Relational Operator21 : RelationalOperator  Operator=<  OutDataTypeStr=boolean  RndMeth=Nearest
  Relational Operator22 : RelationalOperator  Operator=<=  OutDataTypeStr=boolean  RndMeth=Nearest
  Relational Operator23 : RelationalOperator  Operator=<=  OutDataTypeStr=boolean  RndMeth=Nearest
  Relational Operator{4..5,24,34,38} (5 alike) : RelationalOperator  Description=_GWMH9P5.ELK.000_104_  Operator=<  OutDataTypeStr=boolean  RndMeth=Nearest
  Relational Operator26 : RelationalOperator  Description=_GWMH9P5.ELK.000_104_  Operator=<=  OutDataTypeStr=boolean  RndMeth=Nearest
  Relational Operator9 : RelationalOperator  Operator=~=  OutDataTypeStr=boolean  RndMeth=Simplest
  Right : Subsystem
  Selector8 : Selector  Commented=on  InputPortWidth=32  IndexOptions=Index vector (port)  Indices=1  OutputSizes=7
  Terminator1 : Terminator
  Terminator2 : Terminator
  TA_Left_Flag : Outport
  TA_Right_Flag : Outport  Port=2
  wires:
  Relational Operator9/1 -> Logical Operator11/1
  If5/1 -> If Action Threat_Assessment_Left/Action
  [Host_Veh_Width] -> If Action Threat_Assessment_Left/3(Host_Veh_Width)
  [ObjsSelect_Width] -> If Action Threat_Assessment_Left/1(ObjsSelect_Width)
  [left_boundary_lat_rate_filtered] -> If Action Threat_Assessment_Left/4(left_boundary_lat_rate_filtered)
  [ObjsSelect_ID] -> Relational Operator9/1
  Bus Selector1/5 -> [ObjsSelect_LatDist]  "<dyCenter>"
  Logical Operator12/1 -> Logical Operator11/2
  Logical Operator11/1 -> [ELK_Obj_Valid_Flag]
  Bus Selector1/7 -> [ObjsSelect_LatVel]  "<vyRel>"
  [ELK_Left_OC_Obj_Flag] -> If5/1
  [TTC_Calculated] -> If Action Threat_Assessment_Left/2(TTC_Calculated)
  Bus Selector1/4 -> [ObjsSelect_LongiDist]  "<dxCenter>"
  Bus Selector1/2 -> [ObjsSelect_Type]  "<objType>"
  Bus Selector1/1 -> [ObjsSelect_ID]  "<objID>"
  [TA_Right_Flag] -> TA_Right_Flag/1
  [TA_Left_Flag] -> TA_Left_Flag/1
  Bus Selector1/6 -> [ObjsSelect_LongiVel]  "<vxRel>"
  Bus Selector1/3 -> [ObjsSelect_Width]  "<objWidth>"
  Constant72/1 -> Relational Operator9/2
  [ObjsSelect_Width] -> If Action Threat_Assessment_Right/1(ObjsSelect_Width)
  [TTC_Calculated] -> If Action Threat_Assessment_Right/2(TTC_Calculated)
  [right_boundary_lat_rate_filtered] -> If Action Threat_Assessment_Right/4(right_boundary_lat_rate_filtered)
  Merge1/1 -> [TA_Right_Flag_OC]
  If Action Subsystem1/1(Out1) -> Merge1/1
  Constant77/1 -> Relational Operator13/2
  If Action Threat_Assessment_Left/1(TA_Left_Flag) -> Merge/2
  [ELK_Right_OC_Obj_Flag] -> If6/1
  If6/2 -> If Action Subsystem1/Action
  If6/1 -> If Action Threat_Assessment_Right/Action
  Merge/1 -> [TA_Left_Flag_OC]
  Relational Operator13/1 -> Logical Operator12/3
  If5/2 -> If Action Subsystem/Action
  Constant75/1 -> Relational Operator10/2
  Relational Operator12/1 -> Logical Operator12/2
  If Action Subsystem/1(Out) -> Merge/1
  Relational Operator10/1 -> Logical Operator12/1
  [ObjsSelect_Type] -> Relational Operator13/1, Relational Operator12/1, Relational Operator10/1
  [Host_Veh_Width] -> If Action Threat_Assessment_Right/3(Host_Veh_Width)
  If Action Threat_Assessment_Right/1(TA_Right_Flag1) -> Merge1/2
  Constant76/1 -> Relational Operator12/2
  [Objs_all] -> Bus Selector1/1
  From37/1 -> Selector8/2
  [a0_LeftLine] -> Left/1(a0_LeftLine)
  [a1_LeftLine] -> Left/2(a1_LeftLine)
  [right_line_width] -> Right/10(right_line_width)
  [ObjsSelect_LongiDist] -> Right/5(ObjsSelect_LongiDist)
  Right/1(Obj_LatVel_To_RightMkr) -> [Obj_LatVel_To_RightMkr]
  Left/2(Obj_Left_dist_mkr) -> [Obj_Left_dist_mkr]
  [a3_LeftLine] -> Left/4(a3_LeftLine)
  [left_line_width] -> Left/10(left_line_width)
  Left/1(Obj_LatVel_To_LeftMkr) -> [Obj_LatVel_To_LeftMkr]
  [ObjsSelect_LatDist] -> Left/6(ObjsSelect_LatDist)
  [a1_RightLine] -> Right/2(a1_RightLine)
  [Obj_Left_LatDist_Trans] -> If Action Threat_Assessment_Left/6(Obj_Left_LatDist_Trans)
  [ObjsSelect_Width] -> Right/9(ObjsSelect_Width)
  [a2_RightLine] -> Right/3(a2_RightLine)
  [a0_RightLine] -> Right/1(a0_RightLine)
  [ObjsSelect_LatDist] -> Right/6(ObjsSelect_LatDist)
  [ObjsSelect_Width] -> Left/9(ObjsSelect_Width)
  [vyAbsObj] -> Right/8(ObjsSelect_LatVel_abs)
  [vxAbsObj] -> Left/7(ObjsSelect_LongiVel_abs)
  [vxAbsObj] -> Right/7(ObjsSelect_LongiVel_abs)
  [vyAbsObj] -> Left/8(ObjsSelect_LatVel_abs)
  [Obj_LatVel_To_RightMkr] -> If Action Threat_Assessment_Right/5(Obj_LatVel_To_RightMkr)
  Left/3(Obj_Left_LatDist_Trans) -> [Obj_Left_LatDist_Trans]
  [a3_RightLine] -> Right/4(a3_RightLine)
  Right/2(Obj_Right_dist_mkr) -> [Obj_Right_dist_mkr]
  Right/3(Obj_Right_LatDist_Trans) -> [Obj_Right_LatDist_Trans]
  [Obj_Right_LatDist_Trans] -> If Action Threat_Assessment_Right/6(Obj_Right_LatDist_Trans)
  [a2_LeftLine] -> Left/3(a2_LeftLine)
  [ObjsSelect_LongiDist] -> Left/5(ObjsSelect_LongiDist)
  [ELK_Left_OT_Obj_Flag] -> If1/1
  [TA_Right_Flag_OC] -> Logical Operator28/1
  [ELK_Right_Parallel_Obj_Flag] -> Logical Operator28/2
  If1/1 -> If Action Threat_Assessment_Left1/Action
  If Action Subsystem4/1(Out) -> Merge2/1
  [ELK_Right_OT_BSW_Obj_Flag] -> Logical Operator28/3
  [Host_Veh_Width] -> If Action Threat_Assessment_Left1/3(Host_Veh_Width)
  Merge2/1 -> [TA_Left_Flag_OT]
  [ObjsSelect_Width] -> If Action Threat_Assessment_Right1/1(ObjsSelect_Width)
  If Action Threat_Assessment_Right1/1(TA_Right_Flag1) -> Merge3/2
  [ObjsSelect_LatDist] -> If Action Threat_Assessment_Left1/6(Obj_Left_LatDist_Trans)
  Merge3/1 -> [TA_Right_Flag_OT]
  [left_boundary_lat_rate_filtered] -> If Action Threat_Assessment_Left1/4(left_boundary_lat_rate_filtered)
  [ObjsSelect_LatVel] -> If Action Threat_Assessment_Right1/5(Obj_LatVel_To_RightMkr)
  If4/1 -> If Action Threat_Assessment_Right1/Action
  If Action Threat_Assessment_Left1/1(TA_Left_Flag) -> Merge2/2
  [ObjsSelect_LatVel] -> If Action Threat_Assessment_Left1/5(Obj_LatVel_To_LeftMkr)
  [ELK_Right_OT_Obj_Flag] -> If4/1
  [TTC_Calculated] -> If Action Threat_Assessment_Left1/2(TTC_Calculated)
  [ObjsSelect_Width] -> If Action Threat_Assessment_Left1/1(ObjsSelect_Width)
  [TTC_Calculated] -> If Action Threat_Assessment_Right1/2(TTC_Calculated)
  [right_boundary_lat_rate_filtered] -> If Action Threat_Assessment_Right1/4(right_boundary_lat_rate_filtered)
  If4/2 -> If Action Subsystem5/Action
  [Obj_Right_LatDist_Trans] -> If Action Threat_Assessment_Right1/6(Obj_Right_LatDist_Trans)
  [ELK_Left_Parallel_Obj_Flag] -> Logical Operator27/2
  If1/2 -> If Action Subsystem4/Action
  [ELK_Left_OT_BSW_Obj_Flag] -> Logical Operator27/3
  [TA_Left_Flag_OT] -> Logical Operator27/4
  Logical Operator27/1 -> [TA_Left_Flag]
  If Action Subsystem5/1(Out1) -> Merge3/1
  [Host_Veh_Width] -> If Action Threat_Assessment_Right1/3(Host_Veh_Width)
  [TA_Left_Flag_OC] -> Logical Operator27/1
  Logical Operator28/1 -> [TA_Right_Flag]
  [TA_Right_Flag_OT] -> Logical Operator28/4
  Objs_all/1 -> [Objs_all]
  Host_Veh_Width/1 -> [Host_Veh_Width]
  left_boundary_lat_rate_filtered/1 -> [left_boundary_lat_rate_filtered]
  right_boundary_lat_rate_filtered/1 -> [right_boundary_lat_rate_filtered]
  left_line_width/1 -> [left_line_width]
  right_line_width/1 -> [right_line_width]
  a0_LeftLine/1 -> [a0_LeftLine]
  a1_LeftLine/1 -> [a1_LeftLine]
  a2_LeftLine/1 -> [a2_LeftLine]
  a3_LeftLine/1 -> [a3_LeftLine]
  SP_LeftLine/1 -> Terminator2/1
  EP_LeftLine/1 -> [EP_LeftLine]
  a0_RightLine/1 -> [a0_RightLine]
  a1_RightLine/1 -> [a1_RightLine]
  a2_RightLine/1 -> [a2_RightLine]
  a3_RightLine/1 -> [a3_RightLine]
  SP_RightLine/1 -> Terminator1/1
  EP_RightLine/1 -> [EP_RightLine]
  ELK_RightLine_Valid_Flag/1 -> [ELK_RightLine_Valid_Flag]
  ELK_LeftLine_Valid_Flag/1 -> [ELK_LeftLine_Valid_Flag]
  vxAbsObj/1 -> [vxAbsObj]
  vyAbsObj/1 -> [vyAbsObj]
  ForIterator_enum/1 -> [ForIterator_enum]
  TTC_Calculated/1 -> [TTC_Calculated]
  Bus Selector1/8 -> [ObjsSelect_MovingState]  "<movingStatus>"
  [Obj_LatVel_To_LeftMkr] -> If Action Threat_Assessment_Left/5(Obj_LatVel_To_LeftMkr)
  Gain4/1 -> Relational Operator26/2
  [a0_RightLine] -> Relational Operator25/2
  [a0_LeftLine] -> Add/2
  [ELK_Obj_Valid_Flag] -> Logical Operator2/1
  Constant85/1 -> Relational Operator22/2
  [ELK_Obj_Valid_Flag] -> Logical Operator22/1
  Add2/1 -> Relational Operator37/1
  Constant70/1 -> Relational Operator16/2
  Gain5/1 -> Relational Operator34/2
  Add/1 -> Relational Operator15/2
  [ObjsSelect_LongiVel] -> Relational Operator5/1
  [ObjsSelect_LongiDist] -> Relational Operator6/1, Relational Operator7/2
  [ObjsSelect_LongiVel] -> Relational Operator4/1
  [ELK_Obj_Valid_Flag] -> Logical Operator9/1
  Logical Operator9/1 -> [ELK_Left_OC_Obj_Flag]
  Logical Operator8/1 -> Logical Operator1/3
  Relational Operator3/1 -> Logical Operator8/1
  [ObjsSelect_LongiDist] -> Relational Operator2/1, Relational Operator3/2
  Relational Operator1/1 -> Logical Operator7/1
  [ObjsSelect_LongiDist] -> Relational Operator/1, Relational Operator1/2
  Constant62/1 -> Relational Operator1/1
  Constant69/1 -> Relational Operator3/1
  [ELK_Obj_Valid_Flag] -> Logical Operator1/1
  [ELK_RightLine_Valid_Flag] -> Logical Operator1/2
  Relational Operator16/1 -> Logical Operator4/2
  Relational Operator36/1 -> Logical Operator24/2
  Relational Operator7/1 -> Logical Operator3/1
  Relational Operator30/1 -> Logical Operator18/1
  Relational Operator18/1 -> Logical Operator6/2
  [EP_RightLine] -> Relational Operator2/2
  Relational Operator14/1 -> Logical Operator14/1
  Constant91/1 -> Relational Operator31/1
  Relational Operator2/1 -> Logical Operator8/2
  Logical Operator22/1 -> [ELK_Left_OT_Obj_Flag]
  [ObjsSelect_LatDist] -> Relational Operator36/1, Relational Operator40/2
  [ObjsSelect_MovingState] -> Compare To Constant1/1
  [ObjsSelect_MovingState] -> Compare To Constant/1
  Add1/1 -> Relational Operator32/1
  Constant95/1 -> Add3/1
  [ObjsSelect_LongiDist] -> Relational Operator39/1
  Constant94/1 -> Add2/2
  [Host_Veh_Width] -> Gain2/1
  Relational Operator33/1 -> Logical Operator23/2
  Logical Operator4/1 -> Logical Operator2/3
  Constant68/1 -> Relational Operator5/2
  Relational Operator23/1 -> Logical Operator14/2
  [ObjsSelect_MovingState] -> Compare To Constant3/1
  [ObjsSelect_MovingState] -> Compare To Constant2/1
  Relational Operator21/1 -> Logical Operator13/1
  Constant84/1 -> Gain1/1
  Compare To Constant3/1 -> Logical Operator16/5
  [ObjsSelect_LongiDist] -> Relational Operator31/2, Relational Operator27/1
  Logical Operator24/1 -> Logical Operator22/3
  [ObjsSelect_LatDist] -> Relational Operator15/1, Relational Operator30/2
  Compare To Constant2/1 -> Logical Operator15/5
  [a0_RightLine] -> Add1/1
  Relational Operator26/1 -> Logical Operator15/4
  Compare To Constant1/1 -> Logical Operator2/4
  Relational Operator37/1 -> Logical Operator23/1
  Constant83/1 -> Relational Operator18/2
  [ObjsSelect_LongiDist] -> Relational Operator35/1
  Relational Operator17/1 -> Logical Operator10/2
  Constant93/1 -> Gain4/1
  Constant90/1 -> Add1/2
  Relational Operator32/1 -> Logical Operator20/1
  Relational Operator35/1 -> Logical Operator21/2
  [a0_LeftLine] -> Relational Operator30/1
  [ObjsSelect_LongiVel] -> Relational Operator34/1
  Relational Operator/1 -> Logical Operator7/2
  [ObjsSelect_LatDist] -> Relational Operator32/2, Relational Operator25/1
  Logical Operator19/1 -> Logical Operator15/2
  Constant73/1 -> Relational Operator14/1
  Constant87/1 -> Relational Operator29/1
  Logical Operator6/1 -> Logical Operator5/2
  [a0_RightLine] -> Add2/1
  [ELK_Obj_Valid_Flag] -> Logical Operator16/1
  Relational Operator31/1 -> Logical Operator19/1
  Constant92/1 -> Relational Operator27/2
  Constant88/1 -> Relational Operator28/2
  Constant98/1 -> Relational Operator35/2
  Relational Operator25/1 -> Logical Operator20/2
  Relational Operator24/1 -> Logical Operator16/4
  [ObjsSelect_LongiVel] -> Relational Operator24/1
  Logical Operator15/1 -> [ELK_Right_OT_BSW_Obj_Flag]
  Logical Operator2/1 -> [ELK_Left_Parallel_Obj_Flag]
  Gain3/1 -> Relational Operator24/2
  Relational Operator4/1 -> Logical Operator9/4
  Relational Operator6/1 -> Logical Operator3/2
  Relational Operator15/1 -> Logical Operator18/2
  Logical Operator17/1 -> Logical Operator16/2
  Constant86/1 -> Relational Operator23/2
  [Obj_Left_dist_mkr] -> Relational Operator21/2, Relational Operator22/1
  Logical Operator1/1 -> [ELK_Right_OC_Obj_Flag]
  Relational Operator19/1 -> Logical Operator6/1
  Constant81/1 -> Relational Operator21/1
  [ObjsSelect_LatDist] -> Relational Operator16/1, Relational Operator8/2
  Logical Operator7/1 -> Logical Operator9/3
  Relational Operator20/1 -> Logical Operator10/1
  Logical Operator3/1 -> Logical Operator2/2
  [ELK_Obj_Valid_Flag] -> Logical Operator5/1
  [Host_Veh_Width] -> Gain/1
  Relational Operator39/1 -> Logical Operator22/2
  Gain2/1 -> Relational Operator17/2
  Relational Operator22/1 -> Logical Operator13/2
  Compare To Constant/1 -> Logical Operator5/4
  Constant82/1 -> Relational Operator19/1
  [EP_LeftLine] -> Relational Operator/2
  [ObjsSelect_LongiVel] -> Relational Operator38/1
  Logical Operator23/1 -> Logical Operator21/3
  Logical Operator18/1 -> Logical Operator16/3
  Relational Operator28/1 -> Logical Operator17/2
  Relational Operator5/1 -> Logical Operator1/4
  [ObjsSelect_LongiDist] -> Relational Operator19/2, Relational Operator18/1
  Logical Operator16/1 -> [ELK_Left_OT_BSW_Obj_Flag]
  Relational Operator38/1 -> Logical Operator22/4
  Add3/1 -> Relational Operator36/2
  [ObjsSelect_LatDist] -> Relational Operator33/1, Relational Operator37/2
  [ObjsSelect_LongiVel] -> Relational Operator26/1
  Logical Operator14/1 -> Logical Operator1/5
  Gain1/1 -> Relational Operator20/1
  [ObjsSelect_LatDist] -> Relational Operator20/2, Relational Operator17/1
  [a0_LeftLine] -> Add3/2
  Constant101/1 -> Relational Operator40/1
  Relational Operator40/1 -> Logical Operator24/1
  Constant89/1 -> Gain3/1
  [ELK_Obj_Valid_Flag] -> Logical Operator15/1
  [ELK_Obj_Valid_Flag] -> Logical Operator21/1
  Logical Operator20/1 -> Logical Operator15/3
  Logical Operator5/1 -> [ELK_Right_Parallel_Obj_Flag]
  Relational Operator34/1 -> Logical Operator21/4
  Constant96/1 -> Relational Operator39/2
  Logical Operator13/1 -> Logical Operator9/5
  Relational Operator27/1 -> Logical Operator19/2
  Constant97/1 -> Gain5/1
  Relational Operator29/1 -> Logical Operator17/1
  Gain/1 -> Relational Operator8/1
  Gain7/1 -> Relational Operator38/2
  Constant99/1 -> Relational Operator33/2
  Relational Operator8/1 -> Logical Operator4/1
  Constant100/1 -> Gain7/1
  Constant71/1 -> Relational Operator7/1
  [ObjsSelect_LongiDist] -> Relational Operator28/1, Relational Operator29/2
  [Obj_Right_dist_mkr] -> Relational Operator14/2, Relational Operator23/1
  Logical Operator10/1 -> Logical Operator5/3
  [ELK_LeftLine_Valid_Flag] -> Logical Operator9/2
  Constant66/1 -> Relational Operator4/2
  Logical Operator21/1 -> [ELK_Right_OT_Obj_Flag]
  Constant74/1 -> Add/1
  Constant78/1 -> Relational Operator6/2

== system TosAeb/ELK_Objsel/Target_Selection/If Action Subsystem/ObjCoord2VehCoord ==
  dxObj : Inport
  dyObj : Inport  Port=2
  dxDiffObjCoord2VehCoord : Inport  Port=3
  Add : Sum
  dxObj_VehCoord : Outport
  dyObj_VehCoord : Outport  Port=2
  wires:
  dxObj/1 -> [dxObj]
  dyObj/1 -> [dyObj]
  dxDiffObjCoord2VehCoord/1 -> [dxDiffObjCoord2VehCoord]
  [dxObj] -> Add/1
  [dxDiffObjCoord2VehCoord] -> Add/2
  [dyObj] -> dyObj_VehCoord/1
  Add/1 -> [dxObj_VehCoord]
  [dxObj_VehCoord] -> dxObj_VehCoord/1

== system TosAeb/ELK_Objsel/Target_Selection/Subsystem/state_rebuild ==
  State : Inport
  Orientation : Inport  Port=2
  vy : Inport  Port=3
  State_match : MATLAB Function  TreatAsAtomicUnit=on
  obj_state : Outport
  wires:
  State_match/1(obj_state) -> obj_state/1
  State/1 -> State_match/1(state)
  Orientation/1 -> State_match/2(orien)
  vy/1 -> State_match/3(vy)

== system TosAeb/ELK_Objsel/Target_Selection/Subsystem/type_rebuild ==
  Obj_type : Inport
  type_match : MATLAB Function  TreatAsAtomicUnit=on
  ObjType : Outport
  wires:
  Obj_type/1 -> type_match/1(type)
  type_match/1(type_re) -> ObjType/1
